simulink model "TSR_Scenario_002_cs"  (Simulink 7.6)
settings: Solver=ode5  SolverMode=SingleTasking  StartTime=0.0  StopTime=inf  FixedStep=1/100  RelTol=1e-3
PreLoadFcn:
  | check_NUTS_defined;
  | 
  | assignin('base',['model_closed_' bdroot],false);
  | 
  | addpath('../includes_TSR/includes_TSR');
  | 
  | switch version('-release')
  | case {'2010a', '2010b', '2011a'}
  | addpath('../includes_TSR/ML2010b');
  | otherwise
  | addpath('../includes_TSR/ML2011b');
  | end
InitFcn:
  | PreScanExperimentInitFcn(bdroot);
  | 
  | addpath('..\includes_TSR');
StopFcn:
  | PreScanExperimentStopFcn(bdroot)
undefined symbols (not defined in the file): DiscIntNeverNeededParam, InitImageOutputDir, x_ini_VRML, y_ini_VRML, z_ini_VRML
libraries: PreScanSimulinkLibrary x79, simulink x28, PreScanUsefulBlocks x21, simulink_extras x12, simulink_need_slupdate x1
(part 19 of 36)

== system TSR_Scenario_002_cs/BMW_X5_2/VISULightSource_Mux ==
  IndicatorLightsLeft : Inport
  IndicatorLightsRight : Inport  Port=2
  FogLightRearLeft : Inport  Port=3
  FogLightRearRight : Inport  Port=4
  FogLightFrontLeft : Inport  Port=5
  FogLightFrontRight : Inport  Port=6
  MainLightRearLeft : Inport  Port=7
  MainLightRearRight : Inport  Port=8
  MainLightFrontLeft : Inport  Port=9
  MainLightFrontRight : Inport  Port=10
  BrakeLightLeft : Inport  Port=11
  BrakeLightRight : Inport  Port=12
  SubMuxLightSource : Mux  Inputs=12
  Lights : Outport
  wires:
  SubMuxLightSource/1 -> Lights/1
  IndicatorLightsLeft/1 -> SubMuxLightSource/1
  IndicatorLightsRight/1 -> SubMuxLightSource/2
  FogLightRearLeft/1 -> SubMuxLightSource/3
  FogLightRearRight/1 -> SubMuxLightSource/4
  FogLightFrontLeft/1 -> SubMuxLightSource/5
  FogLightFrontRight/1 -> SubMuxLightSource/6
  MainLightRearLeft/1 -> SubMuxLightSource/7
  MainLightRearRight/1 -> SubMuxLightSource/8
  MainLightFrontLeft/1 -> SubMuxLightSource/9
  MainLightFrontRight/1 -> SubMuxLightSource/10
  BrakeLightLeft/1 -> SubMuxLightSource/11
  BrakeLightRight/1 -> SubMuxLightSource/12

== system TSR_Scenario_002_cs/BMW_X5_2/VISUSteer_Mux ==
  Column [deg] : Inport
  Steer [deg] : Inport  Port=2
  Mux2 : Mux  Inputs=2
  Steer : Outport
  wires:
  Column [deg]/1 -> Mux2/2
  Mux2/1 -> Steer/1
  Steer [deg]/1 -> Mux2/1

== system TSR_Scenario_002_cs/BMW_X5_2/VISUWheels_Mux ==
  Steer Angle Front [deg] : Inport  SampleTime=TimeStep
  Steer Angle Rear [deg] : Inport  Port=2  SampleTime=TimeStep
  Velocity [m/s] : Inport  Port=3  SampleTime=TimeStep
  zDisp FL [m] : Inport  Port=4  SampleTime=TimeStep
  zDisp FR [m] : Inport  Port=5  SampleTime=TimeStep
  zDisp RL [m] : Inport  Port=6  SampleTime=TimeStep
  zDisp RR [m] : Inport  Port=7  SampleTime=TimeStep
  Constant{,1..3} (4 alike) : Constant  Value=[0 0]  SampleTime=TimeStep
  Constant{4..7} (4 alike) : Constant  Value=0  SampleTime=TimeStep
  Discrete-Time Integrator : DiscreteIntegrator  SampleTime=TimeStep  ICPrevOutput=DiscIntNeverNeededParam  ICPrevScaledInput=DiscIntNeverNeededParam
  Mux{,1..3} (4 alike) : Mux  Inputs=3
  Mux{4,6..8} (4 alike) : Mux  Inputs=[2 1]
  Mux5 : Mux  Inputs=[3 3 3 3 3 3 3 3]
  Radians to Degrees : simulink_extras/Transformations/Radians to Degrees  SystemSampleTime=-1
  omega=V/Rwheel : Gain  Gain=1/0.29
  Wheels : Outport
  wires:
  Mux7/1 -> Mux5/5  "xyz displacement RL [m]"
  Discrete-Time Integrator/1 -> Mux/2, Mux1/2, Mux2/2, Mux3/2  "roty"
  Radians to Degrees/1 -> Discrete-Time Integrator/1
  Constant7/1 -> Mux3/1  "rotx"
  Constant6/1 -> Mux2/1  "rotx"
  Constant5/1 -> Mux1/1  "rotx"
  Constant4/1 -> Mux/1  "rotx"
  Steer Angle Front [deg]/1 -> Mux1/3, Mux/3  "rotz"
  Steer Angle Rear [deg]/1 -> Mux3/3, Mux2/3  "rotz"
  Mux8/1 -> Mux5/7  "xyz displacement RR [m]"
  Mux6/1 -> Mux5/3  "xyz displacement FR [m]"
  Mux4/1 -> Mux5/1  "xyz displacement FL [m]"
  Constant3/1 -> Mux8/1
  Constant2/1 -> Mux7/1
  Constant1/1 -> Mux6/1
  Constant/1 -> Mux4/1
  zDisp RR [m]/1 -> Mux8/2
  zDisp RL [m]/1 -> Mux7/2
  zDisp FR [m]/1 -> Mux6/2
  zDisp FL [m]/1 -> Mux4/2
  Mux5/1 -> Wheels/1
  omega=V/Rwheel/1 -> Radians to Degrees/1  "rotational speed [rad/s]"
  Velocity [m/s]/1 -> omega=V/Rwheel/1
  Mux1/1 -> Mux5/4  "xyz rotation FR [deg]"
  Mux/1 -> Mux5/2  "xyz rotation FL [deg]"
  Mux3/1 -> Mux5/8  "xyz rotation RR [deg]"
  Mux2/1 -> Mux5/6  "xyz rotation RL [deg]"

== system TSR_Scenario_002_cs/Citroen_C3_1/SELF_Demux ==
  SELF : Inport
  Demux1 : Demux  Outputs=3
  Demux2 : Demux  Outputs=3
  Demux3 : Demux  Outputs=[3 3 1 1]
  Demux4 : Demux  Outputs=3
  Gain : Gain  Gain=180/pi
  Gain1 : Gain  Gain=180/pi
  Selector : Selector  InputPortWidth=3  IndexOptions=Index vector (dialog)  Indices=[3]  OutputSizes=1
  compas converter : Subsystem
  xyz2GPS : PreScanUsefulBlocks/xyz2GPS  CoordinateCount=1  GPS_base=[0.000000 0.000000 0.000]
  x [m] : Outport
  y [m] : Outport  Port=2
  z [m] : Outport  Port=3
  rotx [deg] : Outport  Port=4
  roty [deg] : Outport  Port=5
  rotz [deg] : Outport  Port=6
  GPS lat [deg] : Outport  Port=7
  GPS long [deg] : Outport  Port=8
  GPS alt [m] : Outport  Port=9
  velocity [m/s] : Outport  Port=10
  heading [deg] : Outport  Port=11
  yaw rate [deg/s] : Outport  Port=12
  wires:
  Demux3/4 -> Gain1/1
  Gain1/1 -> yaw rate [deg/s]/1
  Demux3/3 -> velocity [m/s]/1
  compas converter/1(heading [deg]) -> heading [deg]/1
  xyz2GPS/1 -> Demux4/1
  Demux4/3 -> GPS alt [m]/1
  Demux4/2 -> GPS long [deg]/1
  Demux4/1 -> GPS lat [deg]/1
  Demux3/2 -> Gain/1, Selector/1
  Demux3/1 -> xyz2GPS/1, Demux1/1
  SELF/1 -> Demux3/1
  Demux2/3 -> rotz [deg]/1
  Demux2/2 -> roty [deg]/1
  Demux2/1 -> rotx [deg]/1
  Demux1/3 -> z [m]/1
  Demux1/2 -> y [m]/1
  Demux1/1 -> x [m]/1
  Gain/1 -> Demux2/1
  Selector/1 -> compas converter/1(rotz [rad])

== system TSR_Scenario_002_cs/Citroen_C3_1/Trajectories ==
  Traj2State1 : Subsystem  Tag=Traj2State
      settings: DeleteFcn=PreScanBlockDeleteFcn;
      mask Type: PreScan Demux Block:
  Trajectory_11 : Subsystem  Tag=Trajectory  mask(PreScan Source Block: Trajectory){FileName='Trajectory_11.mat', TimeStep=1/100}
      mask prompts: TimeStep "Time Step [s]"
      mask help:
      | The Trajectory block contains the trajectories defined in the PreScan Experiment Editor. The output of the trajectory block is the (vehicle) State.
      |
      | For more information, please click Help.
      mask init: same as TSR_Scenario_002_cs/Actros_1860_1/Trajectories/Trajectory_6
      settings: DeleteFcn=PreScanBlockDeleteFcn;
      mask Help: eval('openHelp(312)')
      mask Display: fprintf(FileName)
  Trajectory_11_Offset : Constant  Tag=TrajectoryOffset  Value="[0  0  0  0  0  0]"
  State : Outport  Tag=TrajOutport
  wires:
  Trajectory_11/1(trajectory) -> Traj2State1/1(Traj Org)
  Trajectory_11_Offset/1 -> Traj2State1/2(Offset)
  Traj2State1/1(state) -> State/1

== system TSR_Scenario_002_cs/Citroen_C3_1/VISULightSource_Mux ==
  IndicatorLightsLeft : Inport
  IndicatorLightsRight : Inport  Port=2
  FogLightFrontLeft : Inport  Port=3
  FogLightFrontRight : Inport  Port=4
  MainLightRearLeft : Inport  Port=5
  MainLightRearRight : Inport  Port=6
  MainLightFrontLeft : Inport  Port=7
  MainLightFrontRight : Inport  Port=8
  BrakeLightLeft : Inport  Port=9
  BrakeLightRight : Inport  Port=10
  SubMuxLightSource : Mux  Inputs=10
  Lights : Outport
  wires:
  SubMuxLightSource/1 -> Lights/1
  IndicatorLightsLeft/1 -> SubMuxLightSource/1
  IndicatorLightsRight/1 -> SubMuxLightSource/2
  FogLightFrontLeft/1 -> SubMuxLightSource/3
  FogLightFrontRight/1 -> SubMuxLightSource/4
  MainLightRearLeft/1 -> SubMuxLightSource/5
  MainLightRearRight/1 -> SubMuxLightSource/6
  MainLightFrontLeft/1 -> SubMuxLightSource/7
  MainLightFrontRight/1 -> SubMuxLightSource/8
  BrakeLightLeft/1 -> SubMuxLightSource/9
  BrakeLightRight/1 -> SubMuxLightSource/10

== system TSR_Scenario_002_cs/Citroen_C3_1/VISUSteer_Mux ==
  Column [deg] : Inport
  Steer [deg] : Inport  Port=2
  Mux2 : Mux  Inputs=2
  Steer : Outport
  wires:
  Column [deg]/1 -> Mux2/2
  Mux2/1 -> Steer/1
  Steer [deg]/1 -> Mux2/1

== system TSR_Scenario_002_cs/Citroen_C3_1/VISUWheels_Mux ==
  Steer Angle Front [deg] : Inport  SampleTime=TimeStep
  Steer Angle Rear [deg] : Inport  Port=2  SampleTime=TimeStep
  Velocity [m/s] : Inport  Port=3  SampleTime=TimeStep
  zDisp FL [m] : Inport  Port=4  SampleTime=TimeStep
  zDisp FR [m] : Inport  Port=5  SampleTime=TimeStep
  zDisp RL [m] : Inport  Port=6  SampleTime=TimeStep
  zDisp RR [m] : Inport  Port=7  SampleTime=TimeStep
  Constant{,1..3} (4 alike) : Constant  Value=[0 0]  SampleTime=TimeStep
  Constant{4..7} (4 alike) : Constant  Value=0  SampleTime=TimeStep
  Discrete-Time Integrator : DiscreteIntegrator  SampleTime=TimeStep  ICPrevOutput=DiscIntNeverNeededParam  ICPrevScaledInput=DiscIntNeverNeededParam
  Mux{,1..3} (4 alike) : Mux  Inputs=3
  Mux{4,6..8} (4 alike) : Mux  Inputs=[2 1]
  Mux5 : Mux  Inputs=[3 3 3 3 3 3 3 3]
  Radians to Degrees : simulink_extras/Transformations/Radians to Degrees  SystemSampleTime=-1
  omega=V/Rwheel : Gain  Gain=1/0.29
  Wheels : Outport
  wires:
  Mux7/1 -> Mux5/5  "xyz displacement RL [m]"
  Discrete-Time Integrator/1 -> Mux/2, Mux1/2, Mux2/2, Mux3/2  "roty"
  Radians to Degrees/1 -> Discrete-Time Integrator/1
  Constant7/1 -> Mux3/1  "rotx"
  Constant6/1 -> Mux2/1  "rotx"
  Constant5/1 -> Mux1/1  "rotx"
  Constant4/1 -> Mux/1  "rotx"
  Steer Angle Front [deg]/1 -> Mux1/3, Mux/3  "rotz"
  Steer Angle Rear [deg]/1 -> Mux3/3, Mux2/3  "rotz"
  Mux8/1 -> Mux5/7  "xyz displacement RR [m]"
  Mux6/1 -> Mux5/3  "xyz displacement FR [m]"
  Mux4/1 -> Mux5/1  "xyz displacement FL [m]"
  Constant3/1 -> Mux8/1
  Constant2/1 -> Mux7/1
  Constant1/1 -> Mux6/1
  Constant/1 -> Mux4/1
  zDisp RR [m]/1 -> Mux8/2
  zDisp RL [m]/1 -> Mux7/2
  zDisp FR [m]/1 -> Mux6/2
  zDisp FL [m]/1 -> Mux4/2
  Mux5/1 -> Wheels/1
  omega=V/Rwheel/1 -> Radians to Degrees/1  "rotational speed [rad/s]"
  Velocity [m/s]/1 -> omega=V/Rwheel/1
  Mux1/1 -> Mux5/4  "xyz rotation FR [deg]"
  Mux/1 -> Mux5/2  "xyz rotation FL [deg]"
  Mux3/1 -> Mux5/8  "xyz rotation RR [deg]"
  Mux2/1 -> Mux5/6  "xyz rotation RL [deg]"

== system TSR_Scenario_002_cs/Citroen_C3_2/SELF_Demux ==
  SELF : Inport
  Demux1 : Demux  Outputs=3
  Demux2 : Demux  Outputs=3
  Demux3 : Demux  Outputs=[3 3 1 1]
  Demux4 : Demux  Outputs=3
  Gain : Gain  Gain=180/pi
  Gain1 : Gain  Gain=180/pi
  Selector : Selector  InputPortWidth=3  IndexOptions=Index vector (dialog)  Indices=[3]  OutputSizes=1
  compas converter : Subsystem
  xyz2GPS : PreScanUsefulBlocks/xyz2GPS  CoordinateCount=1  GPS_base=[0.000000 0.000000 0.000]
  x [m] : Outport
  y [m] : Outport  Port=2
  z [m] : Outport  Port=3
  rotx [deg] : Outport  Port=4
  roty [deg] : Outport  Port=5
  rotz [deg] : Outport  Port=6
  GPS lat [deg] : Outport  Port=7
  GPS long [deg] : Outport  Port=8
  GPS alt [m] : Outport  Port=9
  velocity [m/s] : Outport  Port=10
  heading [deg] : Outport  Port=11
  yaw rate [deg/s] : Outport  Port=12
  wires:
  Demux3/4 -> Gain1/1
  Gain1/1 -> yaw rate [deg/s]/1
  Demux3/3 -> velocity [m/s]/1
  compas converter/1(heading [deg]) -> heading [deg]/1
  xyz2GPS/1 -> Demux4/1
  Demux4/3 -> GPS alt [m]/1
  Demux4/2 -> GPS long [deg]/1
  Demux4/1 -> GPS lat [deg]/1
  Demux3/2 -> Gain/1, Selector/1
  Demux3/1 -> xyz2GPS/1, Demux1/1
  SELF/1 -> Demux3/1
  Demux2/3 -> rotz [deg]/1
  Demux2/2 -> roty [deg]/1
  Demux2/1 -> rotx [deg]/1
  Demux1/3 -> z [m]/1
  Demux1/2 -> y [m]/1
  Demux1/1 -> x [m]/1
  Gain/1 -> Demux2/1
  Selector/1 -> compas converter/1(rotz [rad])

== system TSR_Scenario_002_cs/Citroen_C3_2/Trajectories ==
  Traj2State1 : Subsystem  Tag=Traj2State
      settings: DeleteFcn=PreScanBlockDeleteFcn;
      mask Type: PreScan Demux Block:
  Trajectory_3 : Subsystem  Tag=Trajectory  mask(PreScan Source Block: Trajectory){FileName='Trajectory_3.mat', TimeStep=1/100}
      mask prompts: TimeStep "Time Step [s]"
      mask help:
      | The Trajectory block contains the trajectories defined in the PreScan Experiment Editor. The output of the trajectory block is the (vehicle) State.
      |
      | For more information, please click Help.
      mask init: same as TSR_Scenario_002_cs/Actros_1860_1/Trajectories/Trajectory_6
      settings: DeleteFcn=PreScanBlockDeleteFcn;
      mask Help: eval('openHelp(312)')
      mask Display: fprintf(FileName)
  Trajectory_3_Offset : Constant  Tag=TrajectoryOffset  Value="[0  0  0  0  0  0]"
  State : Outport  Tag=TrajOutport
  wires:
  Trajectory_3/1(trajectory) -> Traj2State1/1(Traj Org)
  Trajectory_3_Offset/1 -> Traj2State1/2(Offset)
  Traj2State1/1(state) -> State/1

== system TSR_Scenario_002_cs/Daf_95_1/SELF_Demux ==
  SELF : Inport
  Demux1 : Demux  Outputs=3
  Demux2 : Demux  Outputs=3
  Demux3 : Demux  Outputs=[3 3 1 1]
  Demux4 : Demux  Outputs=3
  Gain : Gain  Gain=180/pi
  Gain1 : Gain  Gain=180/pi
  Selector : Selector  InputPortWidth=3  IndexOptions=Index vector (dialog)  Indices=[3]  OutputSizes=1
  compas converter : Subsystem
  xyz2GPS : PreScanUsefulBlocks/xyz2GPS  CoordinateCount=1  GPS_base=[0.000000 0.000000 0.000]
  x [m] : Outport
  y [m] : Outport  Port=2
  z [m] : Outport  Port=3
  rotx [deg] : Outport  Port=4
  roty [deg] : Outport  Port=5
  rotz [deg] : Outport  Port=6
  GPS lat [deg] : Outport  Port=7
  GPS long [deg] : Outport  Port=8
  GPS alt [m] : Outport  Port=9
  velocity [m/s] : Outport  Port=10
  heading [deg] : Outport  Port=11
  yaw rate [deg/s] : Outport  Port=12
  wires:
  Demux3/4 -> Gain1/1
  Gain1/1 -> yaw rate [deg/s]/1
  Demux3/3 -> velocity [m/s]/1
  compas converter/1(heading [deg]) -> heading [deg]/1
  xyz2GPS/1 -> Demux4/1
  Demux4/3 -> GPS alt [m]/1
  Demux4/2 -> GPS long [deg]/1
  Demux4/1 -> GPS lat [deg]/1
  Demux3/2 -> Gain/1, Selector/1
  Demux3/1 -> xyz2GPS/1, Demux1/1
  SELF/1 -> Demux3/1
  Demux2/3 -> rotz [deg]/1
  Demux2/2 -> roty [deg]/1
  Demux2/1 -> rotx [deg]/1
  Demux1/3 -> z [m]/1
  Demux1/2 -> y [m]/1
  Demux1/1 -> x [m]/1
  Gain/1 -> Demux2/1
  Selector/1 -> compas converter/1(rotz [rad])

== system TSR_Scenario_002_cs/Daf_95_1/Trajectories ==
  Traj2State1 : Subsystem  Tag=Traj2State
      settings: DeleteFcn=PreScanBlockDeleteFcn;
      mask Type: PreScan Demux Block:
  Trajectory_13 : Subsystem  Tag=Trajectory  mask(PreScan Source Block: Trajectory){FileName='Trajectory_13.mat', TimeStep=1/100}
      mask prompts: TimeStep "Time Step [s]"
      mask help:
      | The Trajectory block contains the trajectories defined in the PreScan Experiment Editor. The output of the trajectory block is the (vehicle) State.
      |
      | For more information, please click Help.
      mask init: same as TSR_Scenario_002_cs/Actros_1860_1/Trajectories/Trajectory_6
      settings: DeleteFcn=PreScanBlockDeleteFcn;
      mask Help: eval('openHelp(312)')
      mask Display: fprintf(FileName)
  Trajectory_13_Offset : Constant  Tag=TrajectoryOffset  Value="[0  0  0  0  0  0]"
  State : Outport  Tag=TrajOutport
  wires:
  Trajectory_13/1(trajectory) -> Traj2State1/1(Traj Org)
  Trajectory_13_Offset/1 -> Traj2State1/2(Offset)
  Traj2State1/1(state) -> State/1

== system TSR_Scenario_002_cs/Fiat_Bravo_1/SELF_Demux ==
  SELF : Inport
  Demux1 : Demux  Outputs=3
  Demux2 : Demux  Outputs=3
  Demux3 : Demux  Outputs=[3 3 1 1]
  Demux4 : Demux  Outputs=3
  Gain : Gain  Gain=180/pi
  Gain1 : Gain  Gain=180/pi
  Selector : Selector  InputPortWidth=3  IndexOptions=Index vector (dialog)  Indices=[3]  OutputSizes=1
  compas converter : Subsystem
  xyz2GPS : PreScanUsefulBlocks/xyz2GPS  CoordinateCount=1  GPS_base=[0.000000 0.000000 0.000]
  x [m] : Outport
  y [m] : Outport  Port=2
  z [m] : Outport  Port=3
  rotx [deg] : Outport  Port=4
  roty [deg] : Outport  Port=5
  rotz [deg] : Outport  Port=6
  GPS lat [deg] : Outport  Port=7
  GPS long [deg] : Outport  Port=8
  GPS alt [m] : Outport  Port=9
  velocity [m/s] : Outport  Port=10
  heading [deg] : Outport  Port=11
  yaw rate [deg/s] : Outport  Port=12
  wires:
  Demux3/4 -> Gain1/1
  Gain1/1 -> yaw rate [deg/s]/1
  Demux3/3 -> velocity [m/s]/1
  compas converter/1(heading [deg]) -> heading [deg]/1
  xyz2GPS/1 -> Demux4/1
  Demux4/3 -> GPS alt [m]/1
  Demux4/2 -> GPS long [deg]/1
  Demux4/1 -> GPS lat [deg]/1
  Demux3/2 -> Gain/1, Selector/1
  Demux3/1 -> xyz2GPS/1, Demux1/1
  SELF/1 -> Demux3/1
  Demux2/3 -> rotz [deg]/1
  Demux2/2 -> roty [deg]/1
  Demux2/1 -> rotx [deg]/1
  Demux1/3 -> z [m]/1
  Demux1/2 -> y [m]/1
  Demux1/1 -> x [m]/1
  Gain/1 -> Demux2/1
  Selector/1 -> compas converter/1(rotz [rad])

== system TSR_Scenario_002_cs/Fiat_Bravo_1/Trajectories ==
  Traj2State1 : Subsystem  Tag=Traj2State
      settings: DeleteFcn=PreScanBlockDeleteFcn;
      mask Type: PreScan Demux Block:
  Trajectory_10 : Subsystem  Tag=Trajectory  mask(PreScan Source Block: Trajectory){FileName='Trajectory_10.mat', TimeStep=1/100}
      mask prompts: TimeStep "Time Step [s]"
      mask help:
      | The Trajectory block contains the trajectories defined in the PreScan Experiment Editor. The output of the trajectory block is the (vehicle) State.
      |
      | For more information, please click Help.
      mask init: same as TSR_Scenario_002_cs/Actros_1860_1/Trajectories/Trajectory_6
      settings: DeleteFcn=PreScanBlockDeleteFcn;
      mask Help: eval('openHelp(312)')
      mask Display: fprintf(FileName)
  Trajectory_10_Offset : Constant  Tag=TrajectoryOffset  Value="[0  0  0  0  0  0]"
  State : Outport  Tag=TrajOutport
  wires:
  Trajectory_10/1(trajectory) -> Traj2State1/1(Traj Org)
  Trajectory_10_Offset/1 -> Traj2State1/2(Offset)
  Traj2State1/1(state) -> State/1

== system TSR_Scenario_002_cs/Fiat_Bravo_1/VISULightSource_Mux ==
  IndicatorLightsLeft : Inport
  IndicatorLightsRight : Inport  Port=2
  FogLightRearLeft : Inport  Port=3
  FogLightFrontLeft : Inport  Port=4
  FogLightFrontRight : Inport  Port=5
  MainLightRearLeft : Inport  Port=6
  MainLightRearRight : Inport  Port=7
  MainLightFrontLeft : Inport  Port=8
  MainLightFrontRight : Inport  Port=9
  BrakeLightLeft : Inport  Port=10
  BrakeLightMiddle : Inport  Port=11
  BrakeLightRight : Inport  Port=12
  ReverseLightRight : Inport  Port=13
  SubMuxLightSource : Mux  Inputs=13
  Lights : Outport
  wires:
  SubMuxLightSource/1 -> Lights/1
  IndicatorLightsLeft/1 -> SubMuxLightSource/1
  IndicatorLightsRight/1 -> SubMuxLightSource/2
  FogLightRearLeft/1 -> SubMuxLightSource/3
  FogLightFrontLeft/1 -> SubMuxLightSource/4
  FogLightFrontRight/1 -> SubMuxLightSource/5
  MainLightRearLeft/1 -> SubMuxLightSource/6
  MainLightRearRight/1 -> SubMuxLightSource/7
  MainLightFrontLeft/1 -> SubMuxLightSource/8
  MainLightFrontRight/1 -> SubMuxLightSource/9
  BrakeLightLeft/1 -> SubMuxLightSource/10
  BrakeLightMiddle/1 -> SubMuxLightSource/11
  BrakeLightRight/1 -> SubMuxLightSource/12
  ReverseLightRight/1 -> SubMuxLightSource/13

== system TSR_Scenario_002_cs/Fiat_Bravo_1/VISUSteer_Mux ==
  Column [deg] : Inport
  Steer [deg] : Inport  Port=2
  Mux2 : Mux  Inputs=2
  Steer : Outport
  wires:
  Column [deg]/1 -> Mux2/2
  Mux2/1 -> Steer/1
  Steer [deg]/1 -> Mux2/1

== system TSR_Scenario_002_cs/Fiat_Bravo_1/VISUWheels_Mux ==
  Steer Angle Front [deg] : Inport  SampleTime=TimeStep
  Steer Angle Rear [deg] : Inport  Port=2  SampleTime=TimeStep
  Velocity [m/s] : Inport  Port=3  SampleTime=TimeStep
  zDisp FL [m] : Inport  Port=4  SampleTime=TimeStep
  zDisp FR [m] : Inport  Port=5  SampleTime=TimeStep
  zDisp RL [m] : Inport  Port=6  SampleTime=TimeStep
  zDisp RR [m] : Inport  Port=7  SampleTime=TimeStep
  Constant{,1..3} (4 alike) : Constant  Value=[0 0]  SampleTime=TimeStep
  Constant{4..7} (4 alike) : Constant  Value=0  SampleTime=TimeStep
  Discrete-Time Integrator : DiscreteIntegrator  SampleTime=TimeStep  ICPrevOutput=DiscIntNeverNeededParam  ICPrevScaledInput=DiscIntNeverNeededParam
  Mux{,1..3} (4 alike) : Mux  Inputs=3
  Mux{4,6..8} (4 alike) : Mux  Inputs=[2 1]
  Mux5 : Mux  Inputs=[3 3 3 3 3 3 3 3]
  Radians to Degrees : simulink_extras/Transformations/Radians to Degrees  SystemSampleTime=-1
  omega=V/Rwheel : Gain  Gain=1/0.29
  Wheels : Outport
  wires:
  Mux7/1 -> Mux5/5  "xyz displacement RL [m]"
  Discrete-Time Integrator/1 -> Mux/2, Mux1/2, Mux2/2, Mux3/2  "roty"
  Radians to Degrees/1 -> Discrete-Time Integrator/1
  Constant7/1 -> Mux3/1  "rotx"
  Constant6/1 -> Mux2/1  "rotx"
  Constant5/1 -> Mux1/1  "rotx"
  Constant4/1 -> Mux/1  "rotx"
  Steer Angle Front [deg]/1 -> Mux1/3, Mux/3  "rotz"
  Steer Angle Rear [deg]/1 -> Mux3/3, Mux2/3  "rotz"
  Mux8/1 -> Mux5/7  "xyz displacement RR [m]"
  Mux6/1 -> Mux5/3  "xyz displacement FR [m]"
  Mux4/1 -> Mux5/1  "xyz displacement FL [m]"
  Constant3/1 -> Mux8/1
  Constant2/1 -> Mux7/1
  Constant1/1 -> Mux6/1
  Constant/1 -> Mux4/1
  zDisp RR [m]/1 -> Mux8/2
  zDisp RL [m]/1 -> Mux7/2
  zDisp FR [m]/1 -> Mux6/2
  zDisp FL [m]/1 -> Mux4/2
  Mux5/1 -> Wheels/1
  omega=V/Rwheel/1 -> Radians to Degrees/1  "rotational speed [rad/s]"
  Velocity [m/s]/1 -> omega=V/Rwheel/1
  Mux1/1 -> Mux5/4  "xyz rotation FR [deg]"
  Mux/1 -> Mux5/2  "xyz rotation FL [deg]"
  Mux3/1 -> Mux5/8  "xyz rotation RR [deg]"
  Mux2/1 -> Mux5/6  "xyz rotation RL [deg]"

== system TSR_Scenario_002_cs/Ford_Fiesta_1/SELF_Demux ==
  SELF : Inport
  Demux1 : Demux  Outputs=3
  Demux2 : Demux  Outputs=3
  Demux3 : Demux  Outputs=[3 3 1 1]
  Demux4 : Demux  Outputs=3
  Gain : Gain  Gain=180/pi
  Gain1 : Gain  Gain=180/pi
  Selector : Selector  InputPortWidth=3  IndexOptions=Index vector (dialog)  Indices=[3]  OutputSizes=1
  compas converter : Subsystem
  xyz2GPS : PreScanUsefulBlocks/xyz2GPS  CoordinateCount=1  GPS_base=[0.000000 0.000000 0.000]
  x [m] : Outport
  y [m] : Outport  Port=2
  z [m] : Outport  Port=3
  rotx [deg] : Outport  Port=4
  roty [deg] : Outport  Port=5
  rotz [deg] : Outport  Port=6
  GPS lat [deg] : Outport  Port=7
  GPS long [deg] : Outport  Port=8
  GPS alt [m] : Outport  Port=9
  velocity [m/s] : Outport  Port=10
  heading [deg] : Outport  Port=11
  yaw rate [deg/s] : Outport  Port=12
  wires:
  Demux3/4 -> Gain1/1
  Gain1/1 -> yaw rate [deg/s]/1
  Demux3/3 -> velocity [m/s]/1
  compas converter/1(heading [deg]) -> heading [deg]/1
  xyz2GPS/1 -> Demux4/1
  Demux4/3 -> GPS alt [m]/1
  Demux4/2 -> GPS long [deg]/1
  Demux4/1 -> GPS lat [deg]/1
  Demux3/2 -> Gain/1, Selector/1
  Demux3/1 -> xyz2GPS/1, Demux1/1
  SELF/1 -> Demux3/1
  Demux2/3 -> rotz [deg]/1
  Demux2/2 -> roty [deg]/1
  Demux2/1 -> rotx [deg]/1
  Demux1/3 -> z [m]/1
  Demux1/2 -> y [m]/1
  Demux1/1 -> x [m]/1
  Gain/1 -> Demux2/1
  Selector/1 -> compas converter/1(rotz [rad])

== system TSR_Scenario_002_cs/Ford_Fiesta_1/Trajectories ==
  Traj2State1 : Subsystem  Tag=Traj2State
      settings: DeleteFcn=PreScanBlockDeleteFcn;
      mask Type: PreScan Demux Block:
  Trajectory_14 : Subsystem  Tag=Trajectory  mask(PreScan Source Block: Trajectory){FileName='Trajectory_14.mat', TimeStep=1/100}
      mask prompts: TimeStep "Time Step [s]"
      mask help:
      | The Trajectory block contains the trajectories defined in the PreScan Experiment Editor. The output of the trajectory block is the (vehicle) State.
      |
      | For more information, please click Help.
      mask init: same as TSR_Scenario_002_cs/Actros_1860_1/Trajectories/Trajectory_6
      settings: DeleteFcn=PreScanBlockDeleteFcn;
      mask Help: eval('openHelp(312)')
      mask Display: fprintf(FileName)
  Trajectory_14_Offset : Constant  Tag=TrajectoryOffset  Value="[0  0  0  0  0  0]"
  State : Outport  Tag=TrajOutport
  wires:
  Trajectory_14/1(trajectory) -> Traj2State1/1(Traj Org)
  Trajectory_14_Offset/1 -> Traj2State1/2(Offset)
  Traj2State1/1(state) -> State/1

== system TSR_Scenario_002_cs/HighwayOverhang_1/VISUMatrixSignLights_Mux ==
  MatrixSign_1 : Inport  PortDimensions=4
  MatrixSign_43 : Inport  Port=2  PortDimensions=4
  Saturation : Saturate  UpperLimit=1  LowerLimit=0
  SubMuxMatrixSignLights : Mux  Inputs=2
  MatrixSignLights : Outport
  wires:
  SubMuxMatrixSignLights/1 -> Saturation/1
  MatrixSign_1/1 -> SubMuxMatrixSignLights/1
  Saturation/1 -> MatrixSignLights/1
  MatrixSign_43/1 -> SubMuxMatrixSignLights/2

== system TSR_Scenario_002_cs/HighwayOverhang_1/VISUMatrixSign_Mux ==
  MatrixSign_1 : Inport
  MatrixSign_43 : Inport  Port=2
  MatrixSign_1_InitialResource : Constant  Value=17
  MatrixSign_1_Switch : Switch  Criteria=u2 ~= 0
  MatrixSign_43_InitialResource : Constant  Value=17
  MatrixSign_43_Switch : Switch  Criteria=u2 ~= 0
  SubMuxMatrixSign : Mux  Inputs=2
  MatrixSigns : Outport
  wires:
  SubMuxMatrixSign/1 -> MatrixSigns/1
  MatrixSign_1/1 -> MatrixSign_1_Switch/1, MatrixSign_1_Switch/2
  MatrixSign_1_InitialResource/1 -> MatrixSign_1_Switch/3
  MatrixSign_1_Switch/1 -> SubMuxMatrixSign/1
  MatrixSign_43/1 -> MatrixSign_43_Switch/1, MatrixSign_43_Switch/2
  MatrixSign_43_InitialResource/1 -> MatrixSign_43_Switch/3
  MatrixSign_43_Switch/1 -> SubMuxMatrixSign/2

== system TSR_Scenario_002_cs/HighwayOverhang_10/VISUMatrixSignLights_Mux ==
  MatrixSign_17 : Inport  PortDimensions=4
  MatrixSign_18 : Inport  Port=2  PortDimensions=4
  Saturation : Saturate  UpperLimit=1  LowerLimit=0
  SubMuxMatrixSignLights : Mux  Inputs=2
  MatrixSignLights : Outport
  wires:
  SubMuxMatrixSignLights/1 -> Saturation/1
  MatrixSign_17/1 -> SubMuxMatrixSignLights/1
  Saturation/1 -> MatrixSignLights/1
  MatrixSign_18/1 -> SubMuxMatrixSignLights/2

== system TSR_Scenario_002_cs/HighwayOverhang_10/VISUMatrixSign_Mux ==
  MatrixSign_17 : Inport
  MatrixSign_18 : Inport  Port=2
  MatrixSign_17_InitialResource : Constant
  MatrixSign_17_Switch : Switch  Criteria=u2 ~= 0
  MatrixSign_18_InitialResource : Constant
  MatrixSign_18_Switch : Switch  Criteria=u2 ~= 0
  SubMuxMatrixSign : Mux  Inputs=2
  MatrixSigns : Outport
  wires:
  SubMuxMatrixSign/1 -> MatrixSigns/1
  MatrixSign_17/1 -> MatrixSign_17_Switch/1, MatrixSign_17_Switch/2
  MatrixSign_17_InitialResource/1 -> MatrixSign_17_Switch/3
  MatrixSign_17_Switch/1 -> SubMuxMatrixSign/1
  MatrixSign_18/1 -> MatrixSign_18_Switch/1, MatrixSign_18_Switch/2
  MatrixSign_18_InitialResource/1 -> MatrixSign_18_Switch/3
  MatrixSign_18_Switch/1 -> SubMuxMatrixSign/2

== system TSR_Scenario_002_cs/HighwayOverhang_11/VISUMatrixSignLights_Mux ==
  MatrixSign_10 : Inport  PortDimensions=4
  MatrixSign_49 : Inport  Port=2  PortDimensions=4
  Saturation : Saturate  UpperLimit=1  LowerLimit=0
  SubMuxMatrixSignLights : Mux  Inputs=2
  MatrixSignLights : Outport
  wires:
  SubMuxMatrixSignLights/1 -> Saturation/1
  MatrixSign_10/1 -> SubMuxMatrixSignLights/1
  Saturation/1 -> MatrixSignLights/1
  MatrixSign_49/1 -> SubMuxMatrixSignLights/2

== system TSR_Scenario_002_cs/HighwayOverhang_11/VISUMatrixSign_Mux ==
  MatrixSign_10 : Inport
  MatrixSign_49 : Inport  Port=2
  MatrixSign_10_InitialResource : Constant  Value=8
  MatrixSign_10_Switch : Switch  Criteria=u2 ~= 0
  MatrixSign_49_InitialResource : Constant  Value=11
  MatrixSign_49_Switch : Switch  Criteria=u2 ~= 0
  SubMuxMatrixSign : Mux  Inputs=2
  MatrixSigns : Outport
  wires:
  SubMuxMatrixSign/1 -> MatrixSigns/1
  MatrixSign_10/1 -> MatrixSign_10_Switch/1, MatrixSign_10_Switch/2
  MatrixSign_10_InitialResource/1 -> MatrixSign_10_Switch/3
  MatrixSign_10_Switch/1 -> SubMuxMatrixSign/1
  MatrixSign_49/1 -> MatrixSign_49_Switch/1, MatrixSign_49_Switch/2
  MatrixSign_49_InitialResource/1 -> MatrixSign_49_Switch/3
  MatrixSign_49_Switch/1 -> SubMuxMatrixSign/2

== system TSR_Scenario_002_cs/HighwayOverhang_12/VISUMatrixSignLights_Mux ==
  MatrixSign_21 : Inport  PortDimensions=4
  MatrixSign_22 : Inport  Port=2  PortDimensions=4
  Saturation : Saturate  UpperLimit=1  LowerLimit=0
  SubMuxMatrixSignLights : Mux  Inputs=2
  MatrixSignLights : Outport
  wires:
  SubMuxMatrixSignLights/1 -> Saturation/1
  MatrixSign_21/1 -> SubMuxMatrixSignLights/1
  Saturation/1 -> MatrixSignLights/1
  MatrixSign_22/1 -> SubMuxMatrixSignLights/2

== system TSR_Scenario_002_cs/HighwayOverhang_12/VISUMatrixSign_Mux ==
  MatrixSign_21 : Inport
  MatrixSign_22 : Inport  Port=2
  MatrixSign_21_InitialResource : Constant
  MatrixSign_21_Switch : Switch  Criteria=u2 ~= 0
  MatrixSign_22_InitialResource : Constant
  MatrixSign_22_Switch : Switch  Criteria=u2 ~= 0
  SubMuxMatrixSign : Mux  Inputs=2
  MatrixSigns : Outport
  wires:
  SubMuxMatrixSign/1 -> MatrixSigns/1
  MatrixSign_21/1 -> MatrixSign_21_Switch/1, MatrixSign_21_Switch/2
  MatrixSign_21_InitialResource/1 -> MatrixSign_21_Switch/3
  MatrixSign_21_Switch/1 -> SubMuxMatrixSign/1
  MatrixSign_22/1 -> MatrixSign_22_Switch/1, MatrixSign_22_Switch/2
  MatrixSign_22_InitialResource/1 -> MatrixSign_22_Switch/3
  MatrixSign_22_Switch/1 -> SubMuxMatrixSign/2

== system TSR_Scenario_002_cs/HighwayOverhang_13/VISUMatrixSignLights_Mux ==
  MatrixSign_3 : Inport  PortDimensions=4
  MatrixSign_44 : Inport  Port=2  PortDimensions=4
  Saturation : Saturate  UpperLimit=1  LowerLimit=0
  SubMuxMatrixSignLights : Mux  Inputs=2
  MatrixSignLights : Outport
  wires:
  SubMuxMatrixSignLights/1 -> Saturation/1
  MatrixSign_3/1 -> SubMuxMatrixSignLights/1
  Saturation/1 -> MatrixSignLights/1
  MatrixSign_44/1 -> SubMuxMatrixSignLights/2

== system TSR_Scenario_002_cs/HighwayOverhang_13/VISUMatrixSign_Mux ==
  MatrixSign_3 : Inport
  MatrixSign_44 : Inport  Port=2
  MatrixSign_3_InitialResource : Constant  Value=16
  MatrixSign_3_Switch : Switch  Criteria=u2 ~= 0
  MatrixSign_44_InitialResource : Constant  Value=16
  MatrixSign_44_Switch : Switch  Criteria=u2 ~= 0
  SubMuxMatrixSign : Mux  Inputs=2
  MatrixSigns : Outport
  wires:
  SubMuxMatrixSign/1 -> MatrixSigns/1
  MatrixSign_3/1 -> MatrixSign_3_Switch/1, MatrixSign_3_Switch/2
  MatrixSign_3_InitialResource/1 -> MatrixSign_3_Switch/3
  MatrixSign_3_Switch/1 -> SubMuxMatrixSign/1
  MatrixSign_44/1 -> MatrixSign_44_Switch/1, MatrixSign_44_Switch/2
  MatrixSign_44_InitialResource/1 -> MatrixSign_44_Switch/3
  MatrixSign_44_Switch/1 -> SubMuxMatrixSign/2

== system TSR_Scenario_002_cs/HighwayOverhang_14/VISUMatrixSignLights_Mux ==
  MatrixSign_27 : Inport  PortDimensions=4
  MatrixSign_28 : Inport  Port=2  PortDimensions=4
  Saturation : Saturate  UpperLimit=1  LowerLimit=0
  SubMuxMatrixSignLights : Mux  Inputs=2
  MatrixSignLights : Outport
  wires:
  SubMuxMatrixSignLights/1 -> Saturation/1
  MatrixSign_27/1 -> SubMuxMatrixSignLights/1
  Saturation/1 -> MatrixSignLights/1
  MatrixSign_28/1 -> SubMuxMatrixSignLights/2

== system TSR_Scenario_002_cs/HighwayOverhang_14/VISUMatrixSign_Mux ==
  MatrixSign_27 : Inport
  MatrixSign_28 : Inport  Port=2
  MatrixSign_27_InitialResource : Constant
  MatrixSign_27_Switch : Switch  Criteria=u2 ~= 0
  MatrixSign_28_InitialResource : Constant
  MatrixSign_28_Switch : Switch  Criteria=u2 ~= 0
  SubMuxMatrixSign : Mux  Inputs=2
  MatrixSigns : Outport
  wires:
  SubMuxMatrixSign/1 -> MatrixSigns/1
  MatrixSign_27/1 -> MatrixSign_27_Switch/1, MatrixSign_27_Switch/2
  MatrixSign_27_InitialResource/1 -> MatrixSign_27_Switch/3
  MatrixSign_27_Switch/1 -> SubMuxMatrixSign/1
  MatrixSign_28/1 -> MatrixSign_28_Switch/1, MatrixSign_28_Switch/2
  MatrixSign_28_InitialResource/1 -> MatrixSign_28_Switch/3
  MatrixSign_28_Switch/1 -> SubMuxMatrixSign/2

== system TSR_Scenario_002_cs/HighwayOverhang_15/VISUMatrixSignLights_Mux ==
  MatrixSign_5 : Inport  PortDimensions=4
  MatrixSign_46 : Inport  Port=2  PortDimensions=4
  Saturation : Saturate  UpperLimit=1  LowerLimit=0
  SubMuxMatrixSignLights : Mux  Inputs=2
  MatrixSignLights : Outport
  wires:
  SubMuxMatrixSignLights/1 -> Saturation/1
  MatrixSign_5/1 -> SubMuxMatrixSignLights/1
  Saturation/1 -> MatrixSignLights/1
  MatrixSign_46/1 -> SubMuxMatrixSignLights/2

== system TSR_Scenario_002_cs/HighwayOverhang_15/VISUMatrixSign_Mux ==
  MatrixSign_5 : Inport
  MatrixSign_46 : Inport  Port=2
  MatrixSign_46_InitialResource : Constant  Value=14
  MatrixSign_46_Switch : Switch  Criteria=u2 ~= 0
  MatrixSign_5_InitialResource : Constant  Value=14
  MatrixSign_5_Switch : Switch  Criteria=u2 ~= 0
  SubMuxMatrixSign : Mux  Inputs=2
  MatrixSigns : Outport
  wires:
  SubMuxMatrixSign/1 -> MatrixSigns/1
  MatrixSign_5/1 -> MatrixSign_5_Switch/1, MatrixSign_5_Switch/2
  MatrixSign_5_InitialResource/1 -> MatrixSign_5_Switch/3
  MatrixSign_5_Switch/1 -> SubMuxMatrixSign/1
  MatrixSign_46/1 -> MatrixSign_46_Switch/1, MatrixSign_46_Switch/2
  MatrixSign_46_InitialResource/1 -> MatrixSign_46_Switch/3
  MatrixSign_46_Switch/1 -> SubMuxMatrixSign/2

== system TSR_Scenario_002_cs/HighwayOverhang_16/VISUMatrixSignLights_Mux ==
  MatrixSign_29 : Inport  PortDimensions=4
  MatrixSign_30 : Inport  Port=2  PortDimensions=4
  Saturation : Saturate  UpperLimit=1  LowerLimit=0
  SubMuxMatrixSignLights : Mux  Inputs=2
  MatrixSignLights : Outport
  wires:
  SubMuxMatrixSignLights/1 -> Saturation/1
  MatrixSign_29/1 -> SubMuxMatrixSignLights/1
  Saturation/1 -> MatrixSignLights/1
  MatrixSign_30/1 -> SubMuxMatrixSignLights/2

== system TSR_Scenario_002_cs/HighwayOverhang_16/VISUMatrixSign_Mux ==
  MatrixSign_29 : Inport
  MatrixSign_30 : Inport  Port=2
  MatrixSign_29_InitialResource : Constant
  MatrixSign_29_Switch : Switch  Criteria=u2 ~= 0
  MatrixSign_30_InitialResource : Constant
  MatrixSign_30_Switch : Switch  Criteria=u2 ~= 0
  SubMuxMatrixSign : Mux  Inputs=2
  MatrixSigns : Outport
  wires:
  SubMuxMatrixSign/1 -> MatrixSigns/1
  MatrixSign_29/1 -> MatrixSign_29_Switch/1, MatrixSign_29_Switch/2
  MatrixSign_29_InitialResource/1 -> MatrixSign_29_Switch/3
  MatrixSign_29_Switch/1 -> SubMuxMatrixSign/1
  MatrixSign_30/1 -> MatrixSign_30_Switch/1, MatrixSign_30_Switch/2
  MatrixSign_30_InitialResource/1 -> MatrixSign_30_Switch/3
  MatrixSign_30_Switch/1 -> SubMuxMatrixSign/2

== system TSR_Scenario_002_cs/HighwayOverhang_17/VISUMatrixSignLights_Mux ==
  MatrixSign_7 : Inport  PortDimensions=4
  MatrixSign_51 : Inport  Port=2  PortDimensions=4
  Saturation : Saturate  UpperLimit=1  LowerLimit=0
  SubMuxMatrixSignLights : Mux  Inputs=2
  MatrixSignLights : Outport
  wires:
  SubMuxMatrixSignLights/1 -> Saturation/1
  MatrixSign_7/1 -> SubMuxMatrixSignLights/1
  Saturation/1 -> MatrixSignLights/1
  MatrixSign_51/1 -> SubMuxMatrixSignLights/2

== system TSR_Scenario_002_cs/HighwayOverhang_17/VISUMatrixSign_Mux ==
  MatrixSign_7 : Inport
  MatrixSign_51 : Inport  Port=2
  MatrixSign_51_InitialResource : Constant  Value=9
  MatrixSign_51_Switch : Switch  Criteria=u2 ~= 0
  MatrixSign_7_InitialResource : Constant  Value=9
  MatrixSign_7_Switch : Switch  Criteria=u2 ~= 0
  SubMuxMatrixSign : Mux  Inputs=2
  MatrixSigns : Outport
  wires:
  SubMuxMatrixSign/1 -> MatrixSigns/1
  MatrixSign_7/1 -> MatrixSign_7_Switch/1, MatrixSign_7_Switch/2
  MatrixSign_7_InitialResource/1 -> MatrixSign_7_Switch/3
  MatrixSign_7_Switch/1 -> SubMuxMatrixSign/1
  MatrixSign_51/1 -> MatrixSign_51_Switch/1, MatrixSign_51_Switch/2
  MatrixSign_51_InitialResource/1 -> MatrixSign_51_Switch/3
  MatrixSign_51_Switch/1 -> SubMuxMatrixSign/2

== system TSR_Scenario_002_cs/HighwayOverhang_18/VISUMatrixSignLights_Mux ==
  MatrixSign_31 : Inport  PortDimensions=4
  MatrixSign_32 : Inport  Port=2  PortDimensions=4
  Saturation : Saturate  UpperLimit=1  LowerLimit=0
  SubMuxMatrixSignLights : Mux  Inputs=2
  MatrixSignLights : Outport
  wires:
  SubMuxMatrixSignLights/1 -> Saturation/1
  MatrixSign_31/1 -> SubMuxMatrixSignLights/1
  Saturation/1 -> MatrixSignLights/1
  MatrixSign_32/1 -> SubMuxMatrixSignLights/2

== system TSR_Scenario_002_cs/HighwayOverhang_18/VISUMatrixSign_Mux ==
  MatrixSign_31 : Inport
  MatrixSign_32 : Inport  Port=2
  MatrixSign_31_InitialResource : Constant
  MatrixSign_31_Switch : Switch  Criteria=u2 ~= 0
  MatrixSign_32_InitialResource : Constant
  MatrixSign_32_Switch : Switch  Criteria=u2 ~= 0
  SubMuxMatrixSign : Mux  Inputs=2
  MatrixSigns : Outport
  wires:
  SubMuxMatrixSign/1 -> MatrixSigns/1
  MatrixSign_31/1 -> MatrixSign_31_Switch/1, MatrixSign_31_Switch/2
  MatrixSign_31_InitialResource/1 -> MatrixSign_31_Switch/3
  MatrixSign_31_Switch/1 -> SubMuxMatrixSign/1
  MatrixSign_32/1 -> MatrixSign_32_Switch/1, MatrixSign_32_Switch/2
  MatrixSign_32_InitialResource/1 -> MatrixSign_32_Switch/3
  MatrixSign_32_Switch/1 -> SubMuxMatrixSign/2

== system TSR_Scenario_002_cs/HighwayOverhang_19/VISUMatrixSignLights_Mux ==
  MatrixSign_9 : Inport  PortDimensions=4
  MatrixSign_52 : Inport  Port=2  PortDimensions=4
  Saturation : Saturate  UpperLimit=1  LowerLimit=0
  SubMuxMatrixSignLights : Mux  Inputs=2
  MatrixSignLights : Outport
  wires:
  SubMuxMatrixSignLights/1 -> Saturation/1
  MatrixSign_9/1 -> SubMuxMatrixSignLights/1
  Saturation/1 -> MatrixSignLights/1
  MatrixSign_52/1 -> SubMuxMatrixSignLights/2

== system TSR_Scenario_002_cs/HighwayOverhang_19/VISUMatrixSign_Mux ==
  MatrixSign_9 : Inport
  MatrixSign_52 : Inport  Port=2
  MatrixSign_52_InitialResource : Constant  Value=8
  MatrixSign_52_Switch : Switch  Criteria=u2 ~= 0
  MatrixSign_9_InitialResource : Constant  Value=8
  MatrixSign_9_Switch : Switch  Criteria=u2 ~= 0
  SubMuxMatrixSign : Mux  Inputs=2
  MatrixSigns : Outport
  wires:
  SubMuxMatrixSign/1 -> MatrixSigns/1
  MatrixSign_9/1 -> MatrixSign_9_Switch/1, MatrixSign_9_Switch/2
  MatrixSign_9_InitialResource/1 -> MatrixSign_9_Switch/3
  MatrixSign_9_Switch/1 -> SubMuxMatrixSign/1
  MatrixSign_52/1 -> MatrixSign_52_Switch/1, MatrixSign_52_Switch/2
  MatrixSign_52_InitialResource/1 -> MatrixSign_52_Switch/3
  MatrixSign_52_Switch/1 -> SubMuxMatrixSign/2

== system TSR_Scenario_002_cs/HighwayOverhang_2/VISUMatrixSignLights_Mux ==
  MatrixSign_14 : Inport  PortDimensions=4
  MatrixSign_2 : Inport  Port=2  PortDimensions=4
  Saturation : Saturate  UpperLimit=1  LowerLimit=0
  SubMuxMatrixSignLights : Mux  Inputs=2
  MatrixSignLights : Outport
  wires:
  SubMuxMatrixSignLights/1 -> Saturation/1
  MatrixSign_14/1 -> SubMuxMatrixSignLights/1
  Saturation/1 -> MatrixSignLights/1
  MatrixSign_2/1 -> SubMuxMatrixSignLights/2

== system TSR_Scenario_002_cs/HighwayOverhang_2/VISUMatrixSign_Mux ==
  MatrixSign_14 : Inport
  MatrixSign_2 : Inport  Port=2
  MatrixSign_14_InitialResource : Constant
  MatrixSign_14_Switch : Switch  Criteria=u2 ~= 0
  MatrixSign_2_InitialResource : Constant
  MatrixSign_2_Switch : Switch  Criteria=u2 ~= 0
  SubMuxMatrixSign : Mux  Inputs=2
  MatrixSigns : Outport
  wires:
  SubMuxMatrixSign/1 -> MatrixSigns/1
  MatrixSign_14/1 -> MatrixSign_14_Switch/1, MatrixSign_14_Switch/2
  MatrixSign_14_InitialResource/1 -> MatrixSign_14_Switch/3
  MatrixSign_14_Switch/1 -> SubMuxMatrixSign/1
  MatrixSign_2/1 -> MatrixSign_2_Switch/1, MatrixSign_2_Switch/2
  MatrixSign_2_InitialResource/1 -> MatrixSign_2_Switch/3
  MatrixSign_2_Switch/1 -> SubMuxMatrixSign/2

== system TSR_Scenario_002_cs/HighwayOverhang_20/VISUMatrixSignLights_Mux ==
  MatrixSign_33 : Inport  PortDimensions=4
  MatrixSign_34 : Inport  Port=2  PortDimensions=4
  Saturation : Saturate  UpperLimit=1  LowerLimit=0
  SubMuxMatrixSignLights : Mux  Inputs=2
  MatrixSignLights : Outport
  wires:
  SubMuxMatrixSignLights/1 -> Saturation/1
  MatrixSign_33/1 -> SubMuxMatrixSignLights/1
  Saturation/1 -> MatrixSignLights/1
  MatrixSign_34/1 -> SubMuxMatrixSignLights/2

== system TSR_Scenario_002_cs/HighwayOverhang_20/VISUMatrixSign_Mux ==
  MatrixSign_33 : Inport
  MatrixSign_34 : Inport  Port=2
  MatrixSign_33_InitialResource : Constant
  MatrixSign_33_Switch : Switch  Criteria=u2 ~= 0
  MatrixSign_34_InitialResource : Constant
  MatrixSign_34_Switch : Switch  Criteria=u2 ~= 0
  SubMuxMatrixSign : Mux  Inputs=2
  MatrixSigns : Outport
  wires:
  SubMuxMatrixSign/1 -> MatrixSigns/1
  MatrixSign_33/1 -> MatrixSign_33_Switch/1, MatrixSign_33_Switch/2
  MatrixSign_33_InitialResource/1 -> MatrixSign_33_Switch/3
  MatrixSign_33_Switch/1 -> SubMuxMatrixSign/1
  MatrixSign_34/1 -> MatrixSign_34_Switch/1, MatrixSign_34_Switch/2
  MatrixSign_34_InitialResource/1 -> MatrixSign_34_Switch/3
  MatrixSign_34_Switch/1 -> SubMuxMatrixSign/2

== system TSR_Scenario_002_cs/HighwayOverhang_21/VISUMatrixSignLights_Mux ==
  MatrixSign_11 : Inport  PortDimensions=4
  MatrixSign_53 : Inport  Port=2  PortDimensions=4
  Saturation : Saturate  UpperLimit=1  LowerLimit=0
  SubMuxMatrixSignLights : Mux  Inputs=2
  MatrixSignLights : Outport
  wires:
  SubMuxMatrixSignLights/1 -> Saturation/1
  MatrixSign_11/1 -> SubMuxMatrixSignLights/1
  Saturation/1 -> MatrixSignLights/1
  MatrixSign_53/1 -> SubMuxMatrixSignLights/2

== system TSR_Scenario_002_cs/HighwayOverhang_21/VISUMatrixSign_Mux ==
  MatrixSign_11 : Inport
  MatrixSign_53 : Inport  Port=2
  MatrixSign_11_InitialResource : Constant  Value=7
  MatrixSign_11_Switch : Switch  Criteria=u2 ~= 0
  MatrixSign_53_InitialResource : Constant  Value=7
  MatrixSign_53_Switch : Switch  Criteria=u2 ~= 0
  SubMuxMatrixSign : Mux  Inputs=2
  MatrixSigns : Outport
  wires:
  SubMuxMatrixSign/1 -> MatrixSigns/1
  MatrixSign_11/1 -> MatrixSign_11_Switch/1, MatrixSign_11_Switch/2
  MatrixSign_11_InitialResource/1 -> MatrixSign_11_Switch/3
  MatrixSign_11_Switch/1 -> SubMuxMatrixSign/1
  MatrixSign_53/1 -> MatrixSign_53_Switch/1, MatrixSign_53_Switch/2
  MatrixSign_53_InitialResource/1 -> MatrixSign_53_Switch/3
  MatrixSign_53_Switch/1 -> SubMuxMatrixSign/2

== system TSR_Scenario_002_cs/HighwayOverhang_22/VISUMatrixSignLights_Mux ==
  MatrixSign_35 : Inport  PortDimensions=4
  MatrixSign_36 : Inport  Port=2  PortDimensions=4
  Saturation : Saturate  UpperLimit=1  LowerLimit=0
  SubMuxMatrixSignLights : Mux  Inputs=2
  MatrixSignLights : Outport
  wires:
  SubMuxMatrixSignLights/1 -> Saturation/1
  MatrixSign_35/1 -> SubMuxMatrixSignLights/1
  Saturation/1 -> MatrixSignLights/1
  MatrixSign_36/1 -> SubMuxMatrixSignLights/2

== system TSR_Scenario_002_cs/HighwayOverhang_22/VISUMatrixSign_Mux ==
  MatrixSign_35 : Inport
  MatrixSign_36 : Inport  Port=2
  MatrixSign_35_InitialResource : Constant
  MatrixSign_35_Switch : Switch  Criteria=u2 ~= 0
  MatrixSign_36_InitialResource : Constant
  MatrixSign_36_Switch : Switch  Criteria=u2 ~= 0
  SubMuxMatrixSign : Mux  Inputs=2
  MatrixSigns : Outport
  wires:
  SubMuxMatrixSign/1 -> MatrixSigns/1
  MatrixSign_35/1 -> MatrixSign_35_Switch/1, MatrixSign_35_Switch/2
  MatrixSign_35_InitialResource/1 -> MatrixSign_35_Switch/3
  MatrixSign_35_Switch/1 -> SubMuxMatrixSign/1
  MatrixSign_36/1 -> MatrixSign_36_Switch/1, MatrixSign_36_Switch/2
  MatrixSign_36_InitialResource/1 -> MatrixSign_36_Switch/3
  MatrixSign_36_Switch/1 -> SubMuxMatrixSign/2

== system TSR_Scenario_002_cs/HighwayOverhang_23/VISUMatrixSignLights_Mux ==
  MatrixSign_13 : Inport  PortDimensions=4
  MatrixSign_54 : Inport  Port=2  PortDimensions=4
  Saturation : Saturate  UpperLimit=1  LowerLimit=0
  SubMuxMatrixSignLights : Mux  Inputs=2
  MatrixSignLights : Outport
  wires:
  SubMuxMatrixSignLights/1 -> Saturation/1
  MatrixSign_13/1 -> SubMuxMatrixSignLights/1
  Saturation/1 -> MatrixSignLights/1
  MatrixSign_54/1 -> SubMuxMatrixSignLights/2

== system TSR_Scenario_002_cs/HighwayOverhang_23/VISUMatrixSign_Mux ==
  MatrixSign_13 : Inport
  MatrixSign_54 : Inport  Port=2
  MatrixSign_13_InitialResource : Constant  Value=6
  MatrixSign_13_Switch : Switch  Criteria=u2 ~= 0
  MatrixSign_54_InitialResource : Constant  Value=6
  MatrixSign_54_Switch : Switch  Criteria=u2 ~= 0
  SubMuxMatrixSign : Mux  Inputs=2
  MatrixSigns : Outport
  wires:
  SubMuxMatrixSign/1 -> MatrixSigns/1
  MatrixSign_13/1 -> MatrixSign_13_Switch/1, MatrixSign_13_Switch/2
  MatrixSign_13_InitialResource/1 -> MatrixSign_13_Switch/3
  MatrixSign_13_Switch/1 -> SubMuxMatrixSign/1
  MatrixSign_54/1 -> MatrixSign_54_Switch/1, MatrixSign_54_Switch/2
  MatrixSign_54_InitialResource/1 -> MatrixSign_54_Switch/3
  MatrixSign_54_Switch/1 -> SubMuxMatrixSign/2

== system TSR_Scenario_002_cs/HighwayOverhang_24/VISUMatrixSignLights_Mux ==
  MatrixSign_25 : Inport  PortDimensions=4
  MatrixSign_55 : Inport  Port=2  PortDimensions=4
  Saturation : Saturate  UpperLimit=1  LowerLimit=0
  SubMuxMatrixSignLights : Mux  Inputs=2
  MatrixSignLights : Outport
  wires:
  SubMuxMatrixSignLights/1 -> Saturation/1
  MatrixSign_25/1 -> SubMuxMatrixSignLights/1
  Saturation/1 -> MatrixSignLights/1
  MatrixSign_55/1 -> SubMuxMatrixSignLights/2

== system TSR_Scenario_002_cs/HighwayOverhang_24/VISUMatrixSign_Mux ==
  MatrixSign_25 : Inport
  MatrixSign_55 : Inport  Port=2
  MatrixSign_25_InitialResource : Constant  Value=5
  MatrixSign_25_Switch : Switch  Criteria=u2 ~= 0
  MatrixSign_55_InitialResource : Constant  Value=5
  MatrixSign_55_Switch : Switch  Criteria=u2 ~= 0
  SubMuxMatrixSign : Mux  Inputs=2
  MatrixSigns : Outport
  wires:
  SubMuxMatrixSign/1 -> MatrixSigns/1
  MatrixSign_25/1 -> MatrixSign_25_Switch/1, MatrixSign_25_Switch/2
  MatrixSign_25_InitialResource/1 -> MatrixSign_25_Switch/3
  MatrixSign_25_Switch/1 -> SubMuxMatrixSign/1
  MatrixSign_55/1 -> MatrixSign_55_Switch/1, MatrixSign_55_Switch/2
  MatrixSign_55_InitialResource/1 -> MatrixSign_55_Switch/3
  MatrixSign_55_Switch/1 -> SubMuxMatrixSign/2

== system TSR_Scenario_002_cs/HighwayOverhang_25/VISUMatrixSignLights_Mux ==
  MatrixSign_26 : Inport  PortDimensions=4
  MatrixSign_56 : Inport  Port=2  PortDimensions=4
  Saturation : Saturate  UpperLimit=1  LowerLimit=0
  SubMuxMatrixSignLights : Mux  Inputs=2
  MatrixSignLights : Outport
  wires:
  SubMuxMatrixSignLights/1 -> Saturation/1
  MatrixSign_26/1 -> SubMuxMatrixSignLights/1
  Saturation/1 -> MatrixSignLights/1
  MatrixSign_56/1 -> SubMuxMatrixSignLights/2

== system TSR_Scenario_002_cs/HighwayOverhang_25/VISUMatrixSign_Mux ==
  MatrixSign_26 : Inport
  MatrixSign_56 : Inport  Port=2
  MatrixSign_26_InitialResource : Constant  Value=4
  MatrixSign_26_Switch : Switch  Criteria=u2 ~= 0
  MatrixSign_56_InitialResource : Constant  Value=4
  MatrixSign_56_Switch : Switch  Criteria=u2 ~= 0
  SubMuxMatrixSign : Mux  Inputs=2
  MatrixSigns : Outport
  wires:
  SubMuxMatrixSign/1 -> MatrixSigns/1
  MatrixSign_26/1 -> MatrixSign_26_Switch/1, MatrixSign_26_Switch/2
  MatrixSign_26_InitialResource/1 -> MatrixSign_26_Switch/3
  MatrixSign_26_Switch/1 -> SubMuxMatrixSign/1
  MatrixSign_56/1 -> MatrixSign_56_Switch/1, MatrixSign_56_Switch/2
  MatrixSign_56_InitialResource/1 -> MatrixSign_56_Switch/3
  MatrixSign_56_Switch/1 -> SubMuxMatrixSign/2

== system TSR_Scenario_002_cs/HighwayOverhang_26/VISUMatrixSignLights_Mux ==
  MatrixSign_37 : Inport  PortDimensions=4
  MatrixSign_38 : Inport  Port=2  PortDimensions=4
  Saturation : Saturate  UpperLimit=1  LowerLimit=0
  SubMuxMatrixSignLights : Mux  Inputs=2
  MatrixSignLights : Outport
  wires:
  SubMuxMatrixSignLights/1 -> Saturation/1
  MatrixSign_37/1 -> SubMuxMatrixSignLights/1
  Saturation/1 -> MatrixSignLights/1
  MatrixSign_38/1 -> SubMuxMatrixSignLights/2

== system TSR_Scenario_002_cs/HighwayOverhang_26/VISUMatrixSign_Mux ==
  MatrixSign_37 : Inport
  MatrixSign_38 : Inport  Port=2
  MatrixSign_37_InitialResource : Constant
  MatrixSign_37_Switch : Switch  Criteria=u2 ~= 0
  MatrixSign_38_InitialResource : Constant
  MatrixSign_38_Switch : Switch  Criteria=u2 ~= 0
  SubMuxMatrixSign : Mux  Inputs=2
  MatrixSigns : Outport
  wires:
  SubMuxMatrixSign/1 -> MatrixSigns/1
  MatrixSign_37/1 -> MatrixSign_37_Switch/1, MatrixSign_37_Switch/2
  MatrixSign_37_InitialResource/1 -> MatrixSign_37_Switch/3
  MatrixSign_37_Switch/1 -> SubMuxMatrixSign/1
  MatrixSign_38/1 -> MatrixSign_38_Switch/1, MatrixSign_38_Switch/2
  MatrixSign_38_InitialResource/1 -> MatrixSign_38_Switch/3
  MatrixSign_38_Switch/1 -> SubMuxMatrixSign/2

== system TSR_Scenario_002_cs/HighwayOverhang_27/VISUMatrixSignLights_Mux ==
  MatrixSign_39 : Inport  PortDimensions=4
  MatrixSign_40 : Inport  Port=2  PortDimensions=4
  Saturation : Saturate  UpperLimit=1  LowerLimit=0
  SubMuxMatrixSignLights : Mux  Inputs=2
  MatrixSignLights : Outport
  wires:
  SubMuxMatrixSignLights/1 -> Saturation/1
  MatrixSign_39/1 -> SubMuxMatrixSignLights/1
  Saturation/1 -> MatrixSignLights/1
  MatrixSign_40/1 -> SubMuxMatrixSignLights/2

== system TSR_Scenario_002_cs/HighwayOverhang_27/VISUMatrixSign_Mux ==
  MatrixSign_39 : Inport
  MatrixSign_40 : Inport  Port=2
  MatrixSign_39_InitialResource : Constant
  MatrixSign_39_Switch : Switch  Criteria=u2 ~= 0
  MatrixSign_40_InitialResource : Constant
  MatrixSign_40_Switch : Switch  Criteria=u2 ~= 0
  SubMuxMatrixSign : Mux  Inputs=2
  MatrixSigns : Outport
  wires:
  SubMuxMatrixSign/1 -> MatrixSigns/1
  MatrixSign_39/1 -> MatrixSign_39_Switch/1, MatrixSign_39_Switch/2
  MatrixSign_39_InitialResource/1 -> MatrixSign_39_Switch/3
  MatrixSign_39_Switch/1 -> SubMuxMatrixSign/1
  MatrixSign_40/1 -> MatrixSign_40_Switch/1, MatrixSign_40_Switch/2
  MatrixSign_40_InitialResource/1 -> MatrixSign_40_Switch/3
  MatrixSign_40_Switch/1 -> SubMuxMatrixSign/2
